simulink model "dss_ideal2_65536ch_32bits_reset_1966mhz_cx"  (R2021a)
settings: Solver=VariableStepAuto  StartTime=0.0  StopTime=10.0  RelTol=1e-3
undefined symbols (not defined in the file): pfb_coeff_gen_calc
libraries: xbsIndex_r4 x57287, casper_library_misc x416, casper_library_delays x120, xps_library x3, simulink x1
(part 251 of 435)

== system dss_ideal2_65536ch_32bits_reset_1966mhz_cx/fft/fft_direct/butterfly2_3/twiddle/coeff_gen/cosin ==
  theta : Inport
  misci : Inport  Port=2
  Constant4 : xbsIndex_r4/Constant  const=0  gui_display_data_type=Fixed-point  arith_type=Unsigned  n_bits=1  bin_pt=0  preci_type=Single  exp_width=8  frac_width=24  explicit_period=on  period=1  equ=P=C  opselect=C  inp2=PCIN>>17  opr=+  inp1=P  carry=CIN  dbl_ovrd=off  block_type=constant
  Constant5 : xbsIndex_r4/Constant  const=0  gui_display_data_type=Fixed-point  arith_type=Unsigned  n_bits=1  bin_pt=0  preci_type=Single  exp_width=8  frac_width=24  explicit_period=on  period=1  equ=P=C  opselect=C  inp2=PCIN>>17  opr=+  inp1=P  carry=CIN  dbl_ovrd=off  block_type=constant
  Delay : xbsIndex_r4/Delay  rst=off  infoeditControl=Selection of Reset will increase slice count due to use of real FFs and instead of SRLs  en=off  latency=bram_latency  reg_retiming=on  dbl_ovrd=off  block_type=delay
  Delay10 : xbsIndex_r4/Delay  rst=off  infoeditControl=Selection of Reset will increase slice count due to use of real FFs and instead of SRLs  en=off  latency=bram_latency  reg_retiming=on  dbl_ovrd=off  block_type=delay
  Delay8 : xbsIndex_r4/Delay  rst=off  infoeditControl=Selection of Reset will increase slice count due to use of real FFs and instead of SRLs  en=off  latency=bram_latency  reg_retiming=on  dbl_ovrd=off  block_type=delay
  ROM : xbsIndex_r4/ROM  depth=2^(12)  initVector=[44423615990 44418897416 44414440986 44409722411 44405003837 44400285263 44395828833 44391110259 44386391684 44381673110 44377216680 44372498106 44367779532 44363060957 44358604527 44353885953 44349167379 44344448805 44339992375 44335273800 44330555226 44326098796 44321380222 44316661648 44312205218 44307486643 44302768069 44298311639 44293593065 44288874491 44284155917 44279699487 44274980913 44270262338 44265805908 44261087334 44256630904 44251912330 44247193756 44242737326 44238018752 44233300178 44228843748 44224125173 44219406599 44214950169 44210231595 44205775165 44201056591 44196338017 44191881587 44187163013 44182706583 44177988009 44173269435 44168813005 44164094431 44159638001 44154919427 44150462997 44145744423 44141025848 44136569418 44131850844 44127394414 44122675840 44118219410 44113500836 44109044406 44104325832 44099607258 44095150828 44090432254 44085975824 44081257250 44076800821 44072082247 44067625817 44062907243 44058450813 44053732239 44049275809 44044557235 44040100805 44035382231 44030925801 44026207227 44021750797 44017032223 44012575793 44008119363 44003400789 43998944359 43994225786 43989769356 43985050782 43980594352 43975875778 43971419348 43966962918 43962244344 43957787914 43953069340 43948612911 43943894337 43939437907 43934981477 43930262903 43925806473 43921087899 43916631469 43912175040 43907456466 43903000036 43898281462 43893825032 43889368602 43884650029 43880193599 43875737169 43871018595 43866562165 43862105735 43857387162 43852930732 43848212158 43843755728 43839299298 43834580725 43830124295 43825667865 43821211435 43816492861 43812036432 43807580002 43802861428 43798404998 43793948568 43789229995 43784773565 43780317135 43775598561 43771142132 43766685702 43762229272 43757510698 43753054269 43748597839 43744141409 43739422836 43734966406 43730509976 43726053546 43721334973 43716878543 43712422113 43707965684 43703247110 43698790680 43694334250 43689877821 43685159247 43680702817 43676246388 43671789958 43667333528 43662614955 43658158525 43653702095 43649245666 43644789236 43640332806 43635614233 43631157803 43626701373 43622244944 43617788514 43613332084 43608613511 43604157081 43599700652 43595244222 43590787792 43586331363 43581612789 43577156359 43572699930 43568243500 43563787071 43559330641 43554874211 43550417782 43545961352 43541242779 43536786349 43532329920 43527873490 43523417060 43518960631 43514504201 43510047772 43505591342 43501134913 43496678483 43492222053 43487503480 43483047050 43478590621 43474134191 43469677762 43465221332 43460764903 43456308473 43451852044 43447395614 43442939185 43438482755 43434026326 43429569896 43425113467 43420657037 43416200608 43411744178 43407287749 43402831319 43398374890 43393918460 43389462031 43385005601 43380549172 43376092742 43371636313 43367179883 43362723454 43358267025 43353810595 43349354166 43344897736 43340441307 43335984877 43331790592 43327334162 43322877733 43318421304 43313964874 43309508445 43305052015 43300595586 43296139157 43291682727 43287226298 43282769868 43278575583 43274119154 43269662724 43265206295 43260749865 43256293436 43251837007 43247380577 43243186292 43238729863 43234273433 43229817004 43225360575 43220904145 43216447716 43212253430 43207797001 43203340572 43198884142 43194427713 43189971284 43185776998 43181320569 43176864140 43172407711 43167951281 43163756996 43159300567 43154844137 43150387708 43145931279 43141736993 43137280564 43132824135 43128367706 43124173420 43119716991 43115260562 43110804132 43106609847 43102153418 43097696989 43093240559 43089046274 43084589845 43080133416 43075676986 43071482701 43067026272 43062569843 43058113413 43053919128 43049462699 43045006270 43040811985 43036355555 43031899126 43027704841 43023248412 43018791983 43014335553 43010141268 43005684839 43001228410 42997034125 42992577695 42988121266 42983926981 42979470552 42975276267 42970819838 42966363408 42962169123 42957712694 42953256265 42949061980 42944605551 42940411265 42935954836 42931498407 42927304122 ...(+45156 chars)]  distributed_mem=Block RAM  rst=off  init_reg=0  en=off  latency=bram_latency  gui_display_data_type=Fixed-point  arith_type=Unsigned  n_bits=n_bits*2  bin_pt=0  preci_type=Single  exp_width=8  frac_width=24  optimize=Speed  use_rpm=on  dbl_ovrd=off  simulation_type=fast_sim  block_type=sprom
  Terminator : Terminator
  Terminator1 : Terminator
  Terminator4 : Terminator
  add_convert0 : Subsystem
  add_convert1 : Subsystem
  assert : xbsIndex_r4/Assert  assert_type=on  type_source=Explicitly  gui_display_data_type=Fixed-point  arith_type=Unsigned  n_bits=12  bin_pt=0  preci_type=Single  exp_width=8  frac_width=24  assert_rate=off  rate_source=Explicitly  period=1  output_port=on  block_type=assert
  c_to_ri : casper_library_misc/c_to_ri  n_bits=n_bits  bin_pt=bin_pt
      settings: SourceProductName=CASPER DSP Blockset
  invert0 : Subsystem  mask{n_bits=n_bits, bin_pt=bin_pt, neg_latency=neg_latency, mux_latency=mux_latency}
      mask prompts: n_bits "bit width"; bin_pt "binary point"; neg_latency "negate latency"; mux_latency "mux latency"
  invert1 : Subsystem  mask{n_bits=n_bits, bin_pt=bin_pt, neg_latency=neg_latency, mux_latency=mux_latency}
      mask prompts: n_bits "bit width"; bin_pt "binary point"; neg_latency "negate latency"; mux_latency "mux latency"
  -sin : Outport
  -cos : Outport  Port=2
  misco : Outport  Port=3
  wires:
  theta/1 -> assert/1
  assert/1 -> add_convert0/1(theta), add_convert1/1(theta)
  Constant4/1 -> add_convert0/2(misci)
  add_convert0/3(misco) -> Terminator/1
  misci/1 -> add_convert1/2(misci)
  add_convert1/3(misco) -> Delay/1
  add_convert0/2(add) -> ROM/1
  add_convert1/2(add) -> Terminator4/1
  ROM/1 -> c_to_ri/1
  add_convert1/1(negate) -> Delay8/1
  add_convert0/1(negate) -> Delay10/1
  Delay10/1 -> invert0/1(negate)
  Constant5/1 -> invert0/3(misci)
  c_to_ri/1 -> invert0/2(in)
  Delay8/1 -> invert1/1(negate)
  c_to_ri/2 -> invert1/2(in)
  invert0/2(misco) -> Terminator1/1
  Delay/1 -> invert1/3(misci)
  invert0/1(out) -> -sin/1
  invert1/1(out) -> -cos/1
  invert1/2(misco) -> misco/1
